simulink model "V2x_TC_IntelligentTrafficSigns_cs"  (Simulink 7.6)
settings: Solver=FixedStepDiscrete  SolverMode=SingleTasking  StartTime=0.0  StopTime=66  FixedStep=1/100  RelTol=1e-3
PreLoadFcn:
  | check_NUTS_defined
  | 
  | assignin('base',['model_closed_' bdroot],false);addpath('../includes_V2x');
InitFcn:
  | PreScanExperimentInitFcn(bdroot)
StopFcn:
  | PreScanExperimentStopFcn(bdroot);
  | 
undefined symbols (not defined in the file or in its folder): acceleration, actorID, actorType, DiscIntNeverNeededParam, GPS_alt, GPS_lat, GPS_long, lightBarInUse, messageID, Newton, publicActorType, Raphson, rotZ, sigbuilder_block, sireneInUse, trafficLightPriority, turnAdvice, velocity, x_ini_VRML, y_ini_VRML, yawRate, z_ini_VRML
libraries: simulink x111, PreScanSimulinkLibrary x7, simulink_extras x4, PreScanUsefulBlocks x3
(part 14 of 16)

== system V2x_TC_IntelligentTrafficSigns_cs/TS_v50_3/Infrastructure CAM generator/set CAM signals ==
  CAM generation signal : Inport
  warning : Inport  Port=2
  messageID : Inport  Port=3
  actorID : Inport  Port=4
  actorType : Inport  Port=5
  trafficLightPriority : Inport  Port=6
  GPS_lat : Inport  Port=7
  GPS_long : Inport  Port=8
  GPS_alt : Inport  Port=9
  rotZ : Inport  Port=10
  Mux : Mux  Inputs=9
  Switch : Switch  Criteria=u2 > Threshold  Threshold=0.5
  Unit Delay : UnitDelay  SampleTime=-1
  CAM_signals : Outport
  wires:
  rotZ/1 -> Mux/9
  GPS_alt/1 -> Mux/8
  GPS_long/1 -> Mux/7
  GPS_lat/1 -> Mux/6
  trafficLightPriority/1 -> Mux/5
  actorType/1 -> Mux/4
  actorID/1 -> Mux/3
  messageID/1 -> Mux/2
  warning/1 -> Mux/1
  Unit Delay/1 -> Switch/3
  Switch/1 -> CAM_signals/1, Unit Delay/1
  Mux/1 -> Switch/1
  CAM generation signal/1 -> Switch/2

== system V2x_TC_IntelligentTrafficSigns_cs/TS_v80_1/Infrastructure CAM generator/CAM generation ==
  Enable : EnablePort  StatesWhenEnabling=reset  PropagateVarSize=During execution
  Add : Sum  Inputs=+-
  Data Store Memory : DataStoreMemory  DataStoreName=CAM_time
  Data Store Read : DataStoreRead  DataStoreName=CAM_time  SampleTime=0.01
  Digital Clock : DigitalClock  SampleTime=0.01
  Merge : Merge
  if : If  IfExpression=u1 >= 1
  no : Subsystem  TreatAsAtomicUnit=on
  yes : Subsystem  TreatAsAtomicUnit=on
  CAN generation signal : Outport  OutputWhenDisabled=reset  InitialOutput=0
  wires:
  if/2 -> no/Action
  Merge/1 -> CAN generation signal/1
  no/1(Out1) -> Merge/2
  yes/1(Out1) -> Merge/1
  if/1 -> yes/Action
  Add/1 -> if/1
  Data Store Read/1 -> Add/2
  Digital Clock/1 -> Add/1

== system V2x_TC_IntelligentTrafficSigns_cs/TS_v80_1/Infrastructure CAM generator/Enabled Subsystem ==
  CAM_sig : Inport
  Enable : EnablePort  PropagateVarSize=During execution
  CAM_signals : Outport  OutputWhenDisabled=reset  InitialOutput=0
  wires:
  CAM_sig/1 -> CAM_signals/1

== system V2x_TC_IntelligentTrafficSigns_cs/TS_v80_1/Infrastructure CAM generator/coder ==
  R : Inport
  O : Inport  Port=2
  G : Inport  Port=3
  Add : Sum  Inputs=+++
  Gain : Gain  Gain=2
  Gain1 : Gain  Gain=3
  trafficLightPriority : Outport
  wires:
  Gain/1 -> Add/2
  Gain1/1 -> Add/3
  R/1 -> Add/1
  O/1 -> Gain/1
  Add/1 -> trafficLightPriority/1
  G/1 -> Gain1/1

== system V2x_TC_IntelligentTrafficSigns_cs/TS_v80_1/Infrastructure CAM generator/impulse generator ==
  CAM_time_step : Inport
  Add : Sum
  Compare To Zero : simulink/Logic and Bit Operations/Compare To Zero  SystemSampleTime=-1  relop=>  LogicOutDataTypeMode=boolean  ZeroCross=off
  Data Type Conversion : DataTypeConversion  OutDataTypeStr=boolean
  Detect Change : simulink/Logic and Bit Operations/Detect Change  SystemSampleTime=-1  vinit=0
  Digital Clock1 : DigitalClock  SampleTime=0.01
  Divide : Product  Inputs=*/
  Gain : Gain  Gain=pi
  Product : Product  RndMeth=Zero
  Trigonometric Function : Trigonometry
  constant : Constant
  experiment time step : Constant  Value=0.01  SampleTime=0
  enable : Outport
  wires:
  CAM_time_step/1 -> Divide/2
  Data Type Conversion/1 -> enable/1
  experiment time step/1 -> Add/2
  Add/1 -> Trigonometric Function/1
  Divide/1 -> Gain/1
  constant/1 -> Divide/1
  Product/1 -> Add/1
  Detect Change/1 -> Data Type Conversion/1
  Compare To Zero/1 -> Detect Change/1
  Trigonometric Function/1 -> Compare To Zero/1
  Gain/1 -> Product/2
  Digital Clock1/1 -> Product/1

== system V2x_TC_IntelligentTrafficSigns_cs/TS_v80_1/Infrastructure CAM generator/impulse generator1 ==
  CAM_time_step : Inport
  Add : Sum
  Compare To Zero : simulink/Logic and Bit Operations/Compare To Zero  SystemSampleTime=-1  relop=>  LogicOutDataTypeMode=boolean  ZeroCross=off
  Data Type Conversion : DataTypeConversion  OutDataTypeStr=boolean
  Detect Change : simulink/Logic and Bit Operations/Detect Change  SystemSampleTime=-1  vinit=0
  Digital Clock1 : DigitalClock  SampleTime=0.01
  Divide : Product  Inputs=*/
  Gain : Gain  Gain=pi
  Product : Product  RndMeth=Zero
  Trigonometric Function : Trigonometry
  constant : Constant
  experiment time step : Constant  Value=0.01  SampleTime=0
  enable : Outport
  wires:
  Digital Clock1/1 -> Product/1
  Gain/1 -> Product/2
  Trigonometric Function/1 -> Compare To Zero/1
  Compare To Zero/1 -> Detect Change/1
  Detect Change/1 -> Data Type Conversion/1
  Product/1 -> Add/1
  constant/1 -> Divide/1
  Divide/1 -> Gain/1
  Add/1 -> Trigonometric Function/1
  experiment time step/1 -> Add/2
  Data Type Conversion/1 -> enable/1
  CAM_time_step/1 -> Divide/2

== system V2x_TC_IntelligentTrafficSigns_cs/TS_v80_1/Infrastructure CAM generator/set CAM signals ==
  CAM generation signal : Inport
  warning : Inport  Port=2
  messageID : Inport  Port=3
  actorID : Inport  Port=4
  actorType : Inport  Port=5
  trafficLightPriority : Inport  Port=6
  GPS_lat : Inport  Port=7
  GPS_long : Inport  Port=8
  GPS_alt : Inport  Port=9
  rotZ : Inport  Port=10
  Mux : Mux  Inputs=9
  Switch : Switch  Criteria=u2 > Threshold  Threshold=0.5
  Unit Delay : UnitDelay  SampleTime=-1
  CAM_signals : Outport
  wires:
  rotZ/1 -> Mux/9
  GPS_alt/1 -> Mux/8
  GPS_long/1 -> Mux/7
  GPS_lat/1 -> Mux/6
  trafficLightPriority/1 -> Mux/5
  actorType/1 -> Mux/4
  actorID/1 -> Mux/3
  messageID/1 -> Mux/2
  warning/1 -> Mux/1
  Unit Delay/1 -> Switch/3
  Switch/1 -> CAM_signals/1, Unit Delay/1
  Mux/1 -> Switch/1
  CAM generation signal/1 -> Switch/2

== system V2x_TC_IntelligentTrafficSigns_cs/TS_v80_3/Infrastructure CAM generator/CAM generation ==
  Enable : EnablePort  StatesWhenEnabling=reset  PropagateVarSize=During execution
  Add : Sum  Inputs=+-
  Data Store Memory : DataStoreMemory  DataStoreName=CAM_time
  Data Store Read : DataStoreRead  DataStoreName=CAM_time  SampleTime=0.01
  Digital Clock : DigitalClock  SampleTime=0.01
  Merge : Merge
  if : If  IfExpression=u1 >= 1
  no : Subsystem  TreatAsAtomicUnit=on
  yes : Subsystem  TreatAsAtomicUnit=on
  CAN generation signal : Outport  OutputWhenDisabled=reset  InitialOutput=0
  wires:
  if/2 -> no/Action
  Merge/1 -> CAN generation signal/1
  no/1(Out1) -> Merge/2
  yes/1(Out1) -> Merge/1
  if/1 -> yes/Action
  Add/1 -> if/1
  Data Store Read/1 -> Add/2
  Digital Clock/1 -> Add/1

== system V2x_TC_IntelligentTrafficSigns_cs/TS_v80_3/Infrastructure CAM generator/Enabled Subsystem ==
  CAM_sig : Inport
  Enable : EnablePort  PropagateVarSize=During execution
  CAM_signals : Outport  OutputWhenDisabled=reset  InitialOutput=0
  wires:
  CAM_sig/1 -> CAM_signals/1

== system V2x_TC_IntelligentTrafficSigns_cs/TS_v80_3/Infrastructure CAM generator/coder ==
  R : Inport
  O : Inport  Port=2
  G : Inport  Port=3
  Add : Sum  Inputs=+++
  Gain : Gain  Gain=2
  Gain1 : Gain  Gain=3
  trafficLightPriority : Outport
  wires:
  Gain/1 -> Add/2
  Gain1/1 -> Add/3
  R/1 -> Add/1
  O/1 -> Gain/1
  Add/1 -> trafficLightPriority/1
  G/1 -> Gain1/1

== system V2x_TC_IntelligentTrafficSigns_cs/TS_v80_3/Infrastructure CAM generator/impulse generator ==
  CAM_time_step : Inport
  Add : Sum
  Compare To Zero : simulink/Logic and Bit Operations/Compare To Zero  SystemSampleTime=-1  relop=>  LogicOutDataTypeMode=boolean  ZeroCross=off
  Data Type Conversion : DataTypeConversion  OutDataTypeStr=boolean
  Detect Change : simulink/Logic and Bit Operations/Detect Change  SystemSampleTime=-1  vinit=0
  Digital Clock1 : DigitalClock  SampleTime=0.01
  Divide : Product  Inputs=*/
  Gain : Gain  Gain=pi
  Product : Product  RndMeth=Zero
  Trigonometric Function : Trigonometry
  constant : Constant
  experiment time step : Constant  Value=0.01  SampleTime=0
  enable : Outport
  wires:
  CAM_time_step/1 -> Divide/2
  Data Type Conversion/1 -> enable/1
  experiment time step/1 -> Add/2
  Add/1 -> Trigonometric Function/1
  Divide/1 -> Gain/1
  constant/1 -> Divide/1
  Product/1 -> Add/1
  Detect Change/1 -> Data Type Conversion/1
  Compare To Zero/1 -> Detect Change/1
  Trigonometric Function/1 -> Compare To Zero/1
  Gain/1 -> Product/2
  Digital Clock1/1 -> Product/1

== system V2x_TC_IntelligentTrafficSigns_cs/TS_v80_3/Infrastructure CAM generator/impulse generator1 ==
  CAM_time_step : Inport
  Add : Sum
  Compare To Zero : simulink/Logic and Bit Operations/Compare To Zero  SystemSampleTime=-1  relop=>  LogicOutDataTypeMode=boolean  ZeroCross=off
  Data Type Conversion : DataTypeConversion  OutDataTypeStr=boolean
  Detect Change : simulink/Logic and Bit Operations/Detect Change  SystemSampleTime=-1  vinit=0
  Digital Clock1 : DigitalClock  SampleTime=0.01
  Divide : Product  Inputs=*/
  Gain : Gain  Gain=pi
  Product : Product  RndMeth=Zero
  Trigonometric Function : Trigonometry
  constant : Constant
  experiment time step : Constant  Value=0.01  SampleTime=0
  enable : Outport
  wires:
  Digital Clock1/1 -> Product/1
  Gain/1 -> Product/2
  Trigonometric Function/1 -> Compare To Zero/1
  Compare To Zero/1 -> Detect Change/1
  Detect Change/1 -> Data Type Conversion/1
  Product/1 -> Add/1
  constant/1 -> Divide/1
  Divide/1 -> Gain/1
  Add/1 -> Trigonometric Function/1
  experiment time step/1 -> Add/2
  Data Type Conversion/1 -> enable/1
  CAM_time_step/1 -> Divide/2

== system V2x_TC_IntelligentTrafficSigns_cs/TS_v80_3/Infrastructure CAM generator/set CAM signals ==
  CAM generation signal : Inport
  warning : Inport  Port=2
  messageID : Inport  Port=3
  actorID : Inport  Port=4
  actorType : Inport  Port=5
  trafficLightPriority : Inport  Port=6
  GPS_lat : Inport  Port=7
  GPS_long : Inport  Port=8
  GPS_alt : Inport  Port=9
  rotZ : Inport  Port=10
  Mux : Mux  Inputs=9
  Switch : Switch  Criteria=u2 > Threshold  Threshold=0.5
  Unit Delay : UnitDelay  SampleTime=-1
  CAM_signals : Outport
  wires:
  rotZ/1 -> Mux/9
  GPS_alt/1 -> Mux/8
  GPS_long/1 -> Mux/7
  GPS_lat/1 -> Mux/6
  trafficLightPriority/1 -> Mux/5
  actorType/1 -> Mux/4
  actorID/1 -> Mux/3
  messageID/1 -> Mux/2
  warning/1 -> Mux/1
  Unit Delay/1 -> Switch/3
  Switch/1 -> CAM_signals/1, Unit Delay/1
  Mux/1 -> Switch/1
  CAM generation signal/1 -> Switch/2

== system V2x_TC_IntelligentTrafficSigns_cs/TS_v80_4/Infrastructure CAM generator/CAM generation ==
  Enable : EnablePort  StatesWhenEnabling=reset  PropagateVarSize=During execution
  Add : Sum  Inputs=+-
  Data Store Memory : DataStoreMemory  DataStoreName=CAM_time
  Data Store Read : DataStoreRead  DataStoreName=CAM_time  SampleTime=0.01
  Digital Clock : DigitalClock  SampleTime=0.01
  Merge : Merge
  if : If  IfExpression=u1 >= 1
  no : Subsystem  TreatAsAtomicUnit=on
  yes : Subsystem  TreatAsAtomicUnit=on
  CAN generation signal : Outport  OutputWhenDisabled=reset  InitialOutput=0
  wires:
  if/2 -> no/Action
  Merge/1 -> CAN generation signal/1
  no/1(Out1) -> Merge/2
  yes/1(Out1) -> Merge/1
  if/1 -> yes/Action
  Add/1 -> if/1
  Data Store Read/1 -> Add/2
  Digital Clock/1 -> Add/1

== system V2x_TC_IntelligentTrafficSigns_cs/TS_v80_4/Infrastructure CAM generator/Enabled Subsystem ==
  CAM_sig : Inport
  Enable : EnablePort  PropagateVarSize=During execution
  CAM_signals : Outport  OutputWhenDisabled=reset  InitialOutput=0
  wires:
  CAM_sig/1 -> CAM_signals/1

== system V2x_TC_IntelligentTrafficSigns_cs/TS_v80_4/Infrastructure CAM generator/coder ==
  R : Inport
  O : Inport  Port=2
  G : Inport  Port=3
  Add : Sum  Inputs=+++
  Gain : Gain  Gain=2
  Gain1 : Gain  Gain=3
  trafficLightPriority : Outport
  wires:
  Gain/1 -> Add/2
  Gain1/1 -> Add/3
  R/1 -> Add/1
  O/1 -> Gain/1
  Add/1 -> trafficLightPriority/1
  G/1 -> Gain1/1

== system V2x_TC_IntelligentTrafficSigns_cs/TS_v80_4/Infrastructure CAM generator/impulse generator ==
  CAM_time_step : Inport
  Add : Sum
  Compare To Zero : simulink/Logic and Bit Operations/Compare To Zero  SystemSampleTime=-1  relop=>  LogicOutDataTypeMode=boolean  ZeroCross=off
  Data Type Conversion : DataTypeConversion  OutDataTypeStr=boolean
  Detect Change : simulink/Logic and Bit Operations/Detect Change  SystemSampleTime=-1  vinit=0
  Digital Clock1 : DigitalClock  SampleTime=0.01
  Divide : Product  Inputs=*/
  Gain : Gain  Gain=pi
  Product : Product  RndMeth=Zero
  Trigonometric Function : Trigonometry
  constant : Constant
  experiment time step : Constant  Value=0.01  SampleTime=0
  enable : Outport
  wires:
  CAM_time_step/1 -> Divide/2
  Data Type Conversion/1 -> enable/1
  experiment time step/1 -> Add/2
  Add/1 -> Trigonometric Function/1
  Divide/1 -> Gain/1
  constant/1 -> Divide/1
  Product/1 -> Add/1
  Detect Change/1 -> Data Type Conversion/1
  Compare To Zero/1 -> Detect Change/1
  Trigonometric Function/1 -> Compare To Zero/1
  Gain/1 -> Product/2
  Digital Clock1/1 -> Product/1

== system V2x_TC_IntelligentTrafficSigns_cs/TS_v80_4/Infrastructure CAM generator/impulse generator1 ==
  CAM_time_step : Inport
  Add : Sum
  Compare To Zero : simulink/Logic and Bit Operations/Compare To Zero  SystemSampleTime=-1  relop=>  LogicOutDataTypeMode=boolean  ZeroCross=off
  Data Type Conversion : DataTypeConversion  OutDataTypeStr=boolean
  Detect Change : simulink/Logic and Bit Operations/Detect Change  SystemSampleTime=-1  vinit=0
  Digital Clock1 : DigitalClock  SampleTime=0.01
  Divide : Product  Inputs=*/
  Gain : Gain  Gain=pi
  Product : Product  RndMeth=Zero
  Trigonometric Function : Trigonometry
  constant : Constant
  experiment time step : Constant  Value=0.01  SampleTime=0
  enable : Outport
  wires:
  Digital Clock1/1 -> Product/1
  Gain/1 -> Product/2
  Trigonometric Function/1 -> Compare To Zero/1
  Compare To Zero/1 -> Detect Change/1
  Detect Change/1 -> Data Type Conversion/1
  Product/1 -> Add/1
  constant/1 -> Divide/1
  Divide/1 -> Gain/1
  Add/1 -> Trigonometric Function/1
  experiment time step/1 -> Add/2
  Data Type Conversion/1 -> enable/1
  CAM_time_step/1 -> Divide/2

== system V2x_TC_IntelligentTrafficSigns_cs/TS_v80_4/Infrastructure CAM generator/set CAM signals ==
  CAM generation signal : Inport
  warning : Inport  Port=2
  messageID : Inport  Port=3
  actorID : Inport  Port=4
  actorType : Inport  Port=5
  trafficLightPriority : Inport  Port=6
  GPS_lat : Inport  Port=7
  GPS_long : Inport  Port=8
  GPS_alt : Inport  Port=9
  rotZ : Inport  Port=10
  Mux : Mux  Inputs=9
  Switch : Switch  Criteria=u2 > Threshold  Threshold=0.5
  Unit Delay : UnitDelay  SampleTime=-1
  CAM_signals : Outport
  wires:
  rotZ/1 -> Mux/9
  GPS_alt/1 -> Mux/8
  GPS_long/1 -> Mux/7
  GPS_lat/1 -> Mux/6
  trafficLightPriority/1 -> Mux/5
  actorType/1 -> Mux/4
  actorID/1 -> Mux/3
  messageID/1 -> Mux/2
  warning/1 -> Mux/1
  Unit Delay/1 -> Switch/3
  Switch/1 -> CAM_signals/1, Unit Delay/1
  Mux/1 -> Switch/1
  CAM generation signal/1 -> Switch/2

== system V2x_TC_IntelligentTrafficSigns_cs/VISUWheels_BMW_X5_1/conv_RotWheelFL/RotMat2AxisAngle ==
  RotMat_Visu : Inport
  Data Type Conversion : DataTypeConversion
  Divide : Product  Inputs=*/
  Gain : Gain  Gain=2
  Mux : Mux  Inputs=3
  Mux1 : Mux  Inputs=2
  Saturation1 : Saturate  UpperLimit=3-0.001  LowerLimit=-1+0.001
  Trigonometric Function : Trigonometry
  U1 : Fcn  Expr=u[6]-u[8]
  U2 : Fcn  Expr=u[7]-u[3]
  U3 : Fcn  Expr=u[2]-u[4]
  U_Phi : Fcn  Expr=u[1]+u[5]+u[9]
  U_Phi1 : Fcn  Expr=acos((u(1)-1)/2)
  U_Phi2 : Fcn  Expr=acos((u(1)-1)/2)
  Angles_Visu : Outport
  wires:
  U_Phi2/1 -> Trigonometric Function/1
  Saturation1/1 -> U_Phi2/1
  U_Phi1/1 -> Data Type Conversion/1
  U_Phi/1 -> Saturation1/1, U_Phi1/1
  Divide/1 -> Mux1/1
  Mux1/1 -> Angles_Visu/1
  Gain/1 -> Divide/2
  Mux/1 -> Divide/1
  U3/1 -> Mux/3
  U2/1 -> Mux/2
  U1/1 -> Mux/1
  Trigonometric Function/1 -> Gain/1
  RotMat_Visu/1 -> U_Phi/1, U3/1, U2/1, U1/1
  Data Type Conversion/1 -> Mux1/2

== system V2x_TC_IntelligentTrafficSigns_cs/VISUWheels_BMW_X5_1/conv_RotWheelFR/RotMat2AxisAngle ==
  RotMat_Visu : Inport
  Data Type Conversion : DataTypeConversion
  Divide : Product  Inputs=*/
  Gain : Gain  Gain=2
  Mux : Mux  Inputs=3
  Mux1 : Mux  Inputs=2
  Saturation1 : Saturate  UpperLimit=3-0.001  LowerLimit=-1+0.001
  Trigonometric Function : Trigonometry
  U1 : Fcn  Expr=u[6]-u[8]
  U2 : Fcn  Expr=u[7]-u[3]
  U3 : Fcn  Expr=u[2]-u[4]
  U_Phi : Fcn  Expr=u[1]+u[5]+u[9]
  U_Phi1 : Fcn  Expr=acos((u(1)-1)/2)
  U_Phi2 : Fcn  Expr=acos((u(1)-1)/2)
  Angles_Visu : Outport
  wires:
  Data Type Conversion/1 -> Mux1/2
  RotMat_Visu/1 -> U1/1, U2/1, U3/1, U_Phi/1
  Trigonometric Function/1 -> Gain/1
  U1/1 -> Mux/1
  U2/1 -> Mux/2
  U3/1 -> Mux/3
  Mux/1 -> Divide/1
  Gain/1 -> Divide/2
  Mux1/1 -> Angles_Visu/1
  Divide/1 -> Mux1/1
  U_Phi/1 -> U_Phi1/1, Saturation1/1
  U_Phi1/1 -> Data Type Conversion/1
  Saturation1/1 -> U_Phi2/1
  U_Phi2/1 -> Trigonometric Function/1

== system V2x_TC_IntelligentTrafficSigns_cs/VISUWheels_BMW_X5_1/conv_RotWheelRL/RotMat2AxisAngle ==
  RotMat_Visu : Inport
  Data Type Conversion : DataTypeConversion
  Divide : Product  Inputs=*/
  Gain : Gain  Gain=2
  Mux : Mux  Inputs=3
  Mux1 : Mux  Inputs=2
  Saturation1 : Saturate  UpperLimit=3-0.001  LowerLimit=-1+0.001
  Trigonometric Function : Trigonometry
  U1 : Fcn  Expr=u[6]-u[8]
  U2 : Fcn  Expr=u[7]-u[3]
  U3 : Fcn  Expr=u[2]-u[4]
  U_Phi : Fcn  Expr=u[1]+u[5]+u[9]
  U_Phi1 : Fcn  Expr=acos((u(1)-1)/2)
  U_Phi2 : Fcn  Expr=acos((u(1)-1)/2)
  Angles_Visu : Outport
  wires:
  Data Type Conversion/1 -> Mux1/2
  RotMat_Visu/1 -> U1/1, U2/1, U3/1, U_Phi/1
  Trigonometric Function/1 -> Gain/1
  U1/1 -> Mux/1
  U2/1 -> Mux/2
  U3/1 -> Mux/3
  Mux/1 -> Divide/1
  Gain/1 -> Divide/2
  Mux1/1 -> Angles_Visu/1
  Divide/1 -> Mux1/1
  U_Phi/1 -> U_Phi1/1, Saturation1/1
  U_Phi1/1 -> Data Type Conversion/1
  Saturation1/1 -> U_Phi2/1
  U_Phi2/1 -> Trigonometric Function/1

== system V2x_TC_IntelligentTrafficSigns_cs/VISUWheels_BMW_X5_1/conv_RotWheelRR/RotMat2AxisAngle ==
  RotMat_Visu : Inport
  Data Type Conversion : DataTypeConversion
  Divide : Product  Inputs=*/
  Gain : Gain  Gain=2
  Mux : Mux  Inputs=3
  Mux1 : Mux  Inputs=2
  Saturation1 : Saturate  UpperLimit=3-0.001  LowerLimit=-1+0.001
  Trigonometric Function : Trigonometry
  U1 : Fcn  Expr=u[6]-u[8]
  U2 : Fcn  Expr=u[7]-u[3]
  U3 : Fcn  Expr=u[2]-u[4]
  U_Phi : Fcn  Expr=u[1]+u[5]+u[9]
  U_Phi1 : Fcn  Expr=acos((u(1)-1)/2)
  U_Phi2 : Fcn  Expr=acos((u(1)-1)/2)
  Angles_Visu : Outport
  wires:
  Data Type Conversion/1 -> Mux1/2
  RotMat_Visu/1 -> U1/1, U2/1, U3/1, U_Phi/1
  Trigonometric Function/1 -> Gain/1
  U1/1 -> Mux/1
  U2/1 -> Mux/2
  U3/1 -> Mux/3
  Mux/1 -> Divide/1
  Gain/1 -> Divide/2
  Mux1/1 -> Angles_Visu/1
  Divide/1 -> Mux1/1
  U_Phi/1 -> U_Phi1/1, Saturation1/1
  U_Phi1/1 -> Data Type Conversion/1
  Saturation1/1 -> U_Phi2/1
  U_Phi2/1 -> Trigonometric Function/1

== system V2x_TC_IntelligentTrafficSigns_cs/VISUWheels_BMW_X5_1/conv_TransWheelFL/pos_ini_Visu ==
  Constant : Constant  Value=x_ini_VRML
  Constant1 : Constant  Value=y_ini_VRML
  Constant2 : Constant  Value=z_ini_VRML
  Mux1 : Mux  Inputs=3
  pos_ini_Visu : Outport
  wires:
  Constant2/1 -> Mux1/3
  Constant1/1 -> Mux1/2
  Mux1/1 -> pos_ini_Visu/1
  Constant/1 -> Mux1/1

== system V2x_TC_IntelligentTrafficSigns_cs/VISUWheels_BMW_X5_1/conv_TransWheelFL/transISO2Visu ==
  transISO : Inport
  Demux : Demux  Outputs=3
  trans_x_Visu : Outport
  trans_y_Visu : Outport  Port=2
  trans_z_Visu : Outport  Port=3
  wires:
  Demux/3 -> trans_z_Visu/1
  transISO/1 -> Demux/1
  Demux/1 -> trans_x_Visu/1
  Demux/2 -> trans_y_Visu/1  "rot_y"

== system V2x_TC_IntelligentTrafficSigns_cs/VISUWheels_BMW_X5_1/conv_TransWheelFR/pos_ini_Visu ==
  Constant : Constant  Value=x_ini_VRML
  Constant1 : Constant  Value=y_ini_VRML
  Constant2 : Constant  Value=z_ini_VRML
  Mux1 : Mux  Inputs=3
  pos_ini_Visu : Outport
  wires:
  Constant/1 -> Mux1/1
  Mux1/1 -> pos_ini_Visu/1
  Constant1/1 -> Mux1/2
  Constant2/1 -> Mux1/3

== system V2x_TC_IntelligentTrafficSigns_cs/VISUWheels_BMW_X5_1/conv_TransWheelFR/transISO2Visu ==
  transISO : Inport
  Demux : Demux  Outputs=3
  trans_x_Visu : Outport
  trans_y_Visu : Outport  Port=2
  trans_z_Visu : Outport  Port=3
  wires:
  Demux/3 -> trans_z_Visu/1
  transISO/1 -> Demux/1
  Demux/1 -> trans_x_Visu/1
  Demux/2 -> trans_y_Visu/1  "rot_y"

== system V2x_TC_IntelligentTrafficSigns_cs/VISUWheels_BMW_X5_1/conv_TransWheelRL/pos_ini_Visu ==
  Constant : Constant  Value=x_ini_VRML
  Constant1 : Constant  Value=y_ini_VRML
  Constant2 : Constant  Value=z_ini_VRML
  Mux1 : Mux  Inputs=3
  pos_ini_Visu : Outport
  wires:
  Constant/1 -> Mux1/1
  Mux1/1 -> pos_ini_Visu/1
  Constant1/1 -> Mux1/2
  Constant2/1 -> Mux1/3

== system V2x_TC_IntelligentTrafficSigns_cs/VISUWheels_BMW_X5_1/conv_TransWheelRL/transISO2Visu ==
  transISO : Inport
  Demux : Demux  Outputs=3
  trans_x_Visu : Outport
  trans_y_Visu : Outport  Port=2
  trans_z_Visu : Outport  Port=3
  wires:
  Demux/3 -> trans_z_Visu/1
  transISO/1 -> Demux/1
  Demux/1 -> trans_x_Visu/1
  Demux/2 -> trans_y_Visu/1  "rot_y"

== system V2x_TC_IntelligentTrafficSigns_cs/VISUWheels_BMW_X5_1/conv_TransWheelRR/pos_ini_Visu ==
  Constant : Constant  Value=x_ini_VRML
  Constant1 : Constant  Value=y_ini_VRML
  Constant2 : Constant  Value=z_ini_VRML
  Mux1 : Mux  Inputs=3
  pos_ini_Visu : Outport
  wires:
  Constant/1 -> Mux1/1
  Mux1/1 -> pos_ini_Visu/1
  Constant1/1 -> Mux1/2
  Constant2/1 -> Mux1/3

== system V2x_TC_IntelligentTrafficSigns_cs/VISUWheels_BMW_X5_1/conv_TransWheelRR/transISO2Visu ==
  transISO : Inport
  Demux : Demux  Outputs=3
  trans_x_Visu : Outport
  trans_y_Visu : Outport  Port=2
  trans_z_Visu : Outport  Port=3
  wires:
  Demux/3 -> trans_z_Visu/1
  transISO/1 -> Demux/1
  Demux/1 -> trans_x_Visu/1
  Demux/2 -> trans_y_Visu/1  "rot_y"

== system V2x_TC_IntelligentTrafficSigns_cs/BMW_X5_1/Car_Rx_1_Demux/compas converter/cart2compas ==
  Angle [deg] : Inport
  Constant : Constant  Value=90
  Constant1 : Constant  Value=360
  Gain : Gain  Gain=1/360
  Gain1 : Gain  Gain=360
  Gain2 : Gain  Gain=-1
  Rounding Function : Rounding
  Sum : Sum  Inputs=|-+
  Sum1 : Sum  Inputs=-+|
  Sum2 : Sum  Inputs=++|
  heading [deg] : Outport
  wires:
  Gain/1 -> Rounding Function/1
  Rounding Function/1 -> Gain1/1
  Gain1/1 -> Sum/1
  Angle [deg]/1 -> Sum1/2
  Sum/1 -> Gain2/1
  Sum1/1 -> Gain/1, Sum/2
  Constant/1 -> Sum1/1
  Gain2/1 -> Sum2/2
  Constant1/1 -> Sum2/1
  Sum2/1 -> heading [deg]/1

== system V2x_TC_IntelligentTrafficSigns_cs/BMW_X5_1/Rx_Noise/For Iterator Subsystem1/con1 ==
  tmp_in : Inport
  i : Inport  Port=2
  CAM_in : Inport  Port=3
  Assignment : Assignment  IndexOptions=Index vector (port)  Indices=1  OutputSizes=1
  Bias1 : Bias  Bias=1
  Selector4 : Selector  NumberOfDimensions=2  IndexOptions=Index vector (port),Index vector (dialog)  Indices=[1 3],3  OutputSizes=1,1
  Selector5 : Selector  IndexOptions=Index vector (port)  Indices=[1 3]  OutputSizes=1
  Switch : Switch  Criteria=u2 ~= 0
  tmp_out : Outport
  wires:
  Selector4/1 -> Switch/2
  Assignment/1 -> Switch/1
  Selector5/1 -> Bias1/1
  Bias1/1 -> Assignment/2
  CAM_in/1 -> Selector4/1
  Switch/1 -> tmp_out/1
  tmp_in/1 -> Assignment/1, Selector5/1, Switch/3
  i/1 -> Selector4/2, Assignment/3, Selector5/2

== system V2x_TC_IntelligentTrafficSigns_cs/BMW_X5_1/Rx_Noise/For Iterator Subsystem1/con2 ==
  CAM_in : Inport
  i : Inport  Port=2
  NoMM : Inport  Port=3
  tmp_in : Inport  Port=4
  Assignment : Assignment  NumberOfDimensions=2  IndexOptions=Index vector (port),Assign all  Indices=1,1  OutputSizes=1,1
  Bias : Bias  Bias=1
  Compare To Zero : simulink/Logic and Bit Operations/Compare To Zero  SystemSampleTime=-1  relop=~=  LogicOutDataTypeMode=boolean
  Compare To Zero1 : simulink/Logic and Bit Operations/Compare To Zero  SystemSampleTime=-1  relop=~=  LogicOutDataTypeMode=boolean
  Constant : Constant  Value=0
  Logical Operator : Logic  AllPortsSameDT=off  OutDataTypeStr=boolean
  Math Function : Math  Operator=mod  SaturateOnIntegerOverflow=on
  Selector4 : Selector  NumberOfDimensions=2  IndexOptions=Index vector (port),Index vector (dialog)  Indices=[1 3],3  OutputSizes=1,1
  Selector5 : Selector  IndexOptions=Index vector (port)  Indices=[1 3]  OutputSizes=1
  Switch : Switch  Criteria=u2 ~= 0
  CAM_o : Outport
  wires:
  Switch/1 -> CAM_o/1
  CAM_in/1 -> Selector4/1, Switch/3, Assignment/1
  Selector4/1 -> Compare To Zero/1
  i/1 -> Selector4/2, Selector5/2, Assignment/3
  Compare To Zero/1 -> Logical Operator/1
  Logical Operator/1 -> Switch/2
  Compare To Zero1/1 -> Logical Operator/2
  Math Function/1 -> Compare To Zero1/1
  tmp_in/1 -> Selector5/1
  Selector5/1 -> Math Function/1
  NoMM/1 -> Bias/1
  Bias/1 -> Math Function/2
  Assignment/1 -> Switch/1
  Constant/1 -> Assignment/2

== system V2x_TC_IntelligentTrafficSigns_cs/BMW_X5_1/SELF_Demux/compas converter/cart2compas ==
  Angle [deg] : Inport
  Constant : Constant  Value=90
  Constant1 : Constant  Value=360
  Gain : Gain  Gain=1/360
  Gain1 : Gain  Gain=360
  Gain2 : Gain  Gain=-1
  Rounding Function : Rounding
  Sum : Sum  Inputs=|-+
  Sum1 : Sum  Inputs=-+|
  Sum2 : Sum  Inputs=++|
  heading [deg] : Outport
  wires:
  Sum2/1 -> heading [deg]/1
  Constant1/1 -> Sum2/1
  Gain2/1 -> Sum2/2
  Constant/1 -> Sum1/1
  Sum1/1 -> Sum/2, Gain/1
  Sum/1 -> Gain2/1
  Angle [deg]/1 -> Sum1/2
  Gain1/1 -> Sum/1
  Rounding Function/1 -> Gain1/1
  Gain/1 -> Rounding Function/1

== system V2x_TC_IntelligentTrafficSigns_cs/BMW_X5_1/Traffic Controller/Intersection warning controller/Enabled Subsystem ==
  crossingType : Inport
  hostTurnAdvice : Inport  Port=2
  hostRotZ : Inport  Port=3
  host GPS lat [deg] : Inport  Port=4
  host GPS long [deg] : Inport  Port=5
  host GPS alt [m] : Inport  Port=6
  GPS_lat : Inport  Port=7
  GPS_long : Inport  Port=8
  GPS_alt : Inport  Port=9
  actorID : Inport  Port=10
  targetVelocity : Inport  Port=11
  turnAdvice : Inport  Port=12
  rotZ : Inport  Port=13
  actorType : Inport  Port=14
  crossing detection range : Inport  Port=15
  Enable : EnablePort  PropagateVarSize=During execution
  Intersection : Subsystem
  sort using ID : Subsystem
  warning left lane : Outport
  warning straight lane : Outport  Port=2
  warning right lane : Outport  Port=3
  wires:
  hostRotZ/1 -> Intersection/1(hostRotZ)
  hostTurnAdvice/1 -> Intersection/2(hostTurnAdvice)
  host GPS lat [deg]/1 -> Intersection/3(host GPS lat [deg])
  host GPS long [deg]/1 -> Intersection/4(host GPS long [deg])
  host GPS alt [m]/1 -> Intersection/5(host GPS alt [m])
  actorID/1 -> sort using ID/1(IDs)
  GPS_lat/1 -> sort using ID/2(in1)
  GPS_long/1 -> sort using ID/3(in2)
  GPS_alt/1 -> sort using ID/4(in3)
  targetVelocity/1 -> sort using ID/5(in4)
  turnAdvice/1 -> sort using ID/6(in5)
  rotZ/1 -> sort using ID/7(in6)
  actorType/1 -> sort using ID/8(in7)
  crossingType/1 -> sort using ID/9(in8)
  sort using ID/1(Out1) -> Intersection/6(GPS_lat)
  sort using ID/2(Out2) -> Intersection/7(GPS_long)
  sort using ID/3(Out3) -> Intersection/8(GPS_alt)
  sort using ID/4(Out4) -> Intersection/9(targetVelocity)
  sort using ID/5(Out5) -> Intersection/10(turnAdvice)
  sort using ID/6(Out6) -> Intersection/11(rotZ)
  sort using ID/7(Out7) -> Intersection/12(actorType)
  sort using ID/8(Out8) -> Intersection/13(crossingType)
  Intersection/1(warning left lane) -> warning left lane/1
  Intersection/2(warning straight lane) -> warning straight lane/1
  Intersection/3(warning right lane) -> warning right lane/1
  crossing detection range/1 -> Intersection/14(crossing detection range)

== system V2x_TC_IntelligentTrafficSigns_cs/BMW_X5_1/Traffic Controller/Road accident detection controller/Compute distance to road accident ==
  conditions : Inport
  host_GPS lat [deg] : Inport  Port=2
  host_GPS lat [deg]1 : Inport  Port=3
  host_GPS alt [m] : Inport  Port=4
  GPS lat [deg] : Inport  Port=5
  GPS long [deg] : Inport  Port=6
  GPS alt [deg] : Inport  Port=7
  Enable : EnablePort  ShowOutputPort=on
  If : If  IfExpression=u1
  If Action Subsystem : Subsystem  TreatAsAtomicUnit=on
  If Action Subsystem1 : Subsystem  TreatAsAtomicUnit=on
  Merge : Merge
  RA_warning : Outport  OutputWhenDisabled=reset  InitialOutput=0
  RA_distance : Outport  Port=2  OutputWhenDisabled=reset  InitialOutput=0
  wires:
  GPS alt [deg]/1 -> If Action Subsystem/4(GPS alt1)
  If/2 -> If Action Subsystem1/Action
  If Action Subsystem1/1(distance) -> Merge/2
  If Action Subsystem/1(distance) -> Merge/1
  GPS long [deg]/1 -> If Action Subsystem/3(GPS long1)
  GPS lat [deg]/1 -> If Action Subsystem/2(GPS lat1)
  If/1 -> If Action Subsystem/Action
  host_GPS lat [deg]1/1 -> If Action Subsystem/6(host_GPS_long)
  host_GPS lat [deg]/1 -> If Action Subsystem/5(host_GPS_lat)
  host_GPS alt [m]/1 -> If Action Subsystem/7(host_GPS_alt)
  Enable/1 -> If/1, RA_warning/1
  conditions/1 -> If Action Subsystem/1(conditions)
  Merge/1 -> RA_distance/1

== system V2x_TC_IntelligentTrafficSigns_cs/BMW_X5_1/Traffic Controller/Road accident detection controller/distance hold ==
  dist : Inport
  Constant : Constant  Value=0
  Discrete FIR Filter : DiscreteFir  NumCoeffs=0.1 * ones(1,10)
  Memory : Memory
  Switch : Switch  Criteria=u2 > Threshold
  Switch1 : Switch  Criteria=u2 ~= 0
  distance : Outport
  wires:
  dist/1 -> Switch/2, Switch/1, Discrete FIR Filter/1
  Switch/1 -> Memory/1, Switch1/1
  Memory/1 -> Switch/3
  Discrete FIR Filter/1 -> Switch1/2
  Constant/1 -> Switch1/3
  Switch1/1 -> distance/1

== system V2x_TC_IntelligentTrafficSigns_cs/BMW_X5_1/Traffic Controller/Road accident detection controller/warning hold ==
  warn : Inport
  Constant : Constant  Value=0
  Discrete FIR Filter : DiscreteFir  NumCoeffs=0.1 * ones(1,10)
  Memory : Memory
  Switch : Switch  Criteria=u2 > Threshold
  Switch1 : Switch  Criteria=u2 ~= 0
  warning : Outport
  wires:
  warn/1 -> Switch/2, Switch/1, Discrete FIR Filter/1
  Switch/1 -> Memory/1, Switch1/1
  Memory/1 -> Switch/3
  Discrete FIR Filter/1 -> Switch1/2
  Constant/1 -> Switch1/3
  Switch1/1 -> warning/1

== system V2x_TC_IntelligentTrafficSigns_cs/BMW_X5_1/Traffic Controller/Traffic signs and  traffic lights controller/Enabled Subsystem ==
  trafficSignType : Inport
  trafficLightsType : Inport  Port=2
  hostRotZ : Inport  Port=3
  hostVelocity : Inport  Port=4
  messageID : Inport  Port=5
  trafficLightPriority : Inport  Port=6
  rotZ : Inport  Port=7
  host GPS lat [deg] : Inport  Port=8
  host GPS long [deg] : Inport  Port=9
  host GPS alt [m] : Inport  Port=10
  GPS_lat : Inport  Port=11
  GPS_long : Inport  Port=12
  GPS_alt : Inport  Port=13
  Enable : EnablePort  StatesWhenEnabling=reset  PropagateVarSize=During execution
  GPS to XYZ difference (vectorized) : Subsystem
  Logical Operator : Logic  AllPortsSameDT=off  OutDataTypeStr=boolean
  Logical Operator1 : Logic  AllPortsSameDT=off  OutDataTypeStr=boolean
  Logical Operator2 : Logic  Inputs=4  AllPortsSameDT=off  OutDataTypeStr=boolean
  distance condition : Subsystem
  heading condition : Subsystem
  hostVelocity1 : InportShadow  Port=4
  major object selection lights : Subsystem
  major object selection signs : Subsystem
  orientation condition : Subsystem
  right road side condition : Subsystem
  signsWarning : Outport
  signsMessage : Outport  Port=2  InitialOutput=0
  lightsWarning : Outport  Port=3
  ligtsMessage : Outport  Port=4  InitialOutput=0
  ligtsPriority : Outport  Port=5  InitialOutput=0
  wires:
  GPS to XYZ difference (vectorized)/3(dY) -> right road side condition/3(dY), orientation condition/3(dY)
  right road side condition/1(orient_cond) -> Logical Operator2/4
  GPS to XYZ difference (vectorized)/1(dist_sq) -> distance condition/1(distance_squared)
  GPS to XYZ difference (vectorized)/2(dX) -> orientation condition/2(dX), right road side condition/2(dX)
  GPS to XYZ difference (vectorized)/4(dZ) -> orientation condition/4(dZ)
  GPS_alt/1 -> GPS to XYZ difference (vectorized)/6(alt)
  orientation condition/1(orient_cond) -> Logical Operator2/3
  GPS_long/1 -> GPS to XYZ difference (vectorized)/5(long)
  GPS_lat/1 -> GPS to XYZ difference (vectorized)/4(lat)
  host GPS alt [m]/1 -> GPS to XYZ difference (vectorized)/3(hostAlt)
  host GPS long [deg]/1 -> GPS to XYZ difference (vectorized)/2(hostLong)
  host GPS lat [deg]/1 -> GPS to XYZ difference (vectorized)/1(hostLat)
  hostVelocity1/1 -> major object selection signs/2(hostVelocity)
  major object selection lights/3(Priority) -> ligtsPriority/1
  major object selection lights/2(MessageID) -> ligtsMessage/1
  major object selection lights/1(Warning) -> lightsWarning/1
  trafficLightPriority/1 -> major object selection lights/3(traffic lights priorities)
  Logical Operator1/1 -> major object selection lights/1(conditions)
  major object selection signs/2(MessageID) -> signsMessage/1
  major object selection signs/1(Warning) -> signsWarning/1
  messageID/1 -> major object selection lights/2(messagesID), major object selection signs/3(messagesID)
  Logical Operator/1 -> major object selection signs/1(conditions)
  trafficLightsType/1 -> Logical Operator1/2
  trafficSignType/1 -> Logical Operator/2
  Logical Operator2/1 -> Logical Operator1/1, Logical Operator/1
  heading condition/1(head_cond) -> Logical Operator2/2
  distance condition/1(dist_cond) -> Logical Operator2/1
  hostRotZ/1 -> orientation condition/1(hostRotZ), right road side condition/1(hostRotZ), heading condition/1(hostRotZ)
  hostVelocity/1 -> distance condition/2(hostVelocity)
  rotZ/1 -> heading condition/2(rotZ)

== system V2x_TC_IntelligentTrafficSigns_cs/BMW_X5_1/Trajectories/ElapsedTrack1/MuxState ==
  xyzPos_[m] : Inport
  RPYAngle_[rad] : Inport  Port=2
  Demux : Demux  Outputs=3
  Discrete Derivative : simulink/Discrete/Discrete Derivative  SystemSampleTime=-1  gainval=1.0  ICPrevScaledInput=0.0  OutMin=[]  OutMax=[]  OutDataType=fixdt(1, 16)  OutScaling=2^-10  DoSatur=off
  Mux : Mux  Inputs=3
  Mux1 : Mux  Inputs=3
  Substitute 90 & 270 deg (and its plurals) : Subsystem
  State : Outport
  wires:
  Mux1/1 -> Mux/3
  Discrete Derivative/1 -> Mux/2
  RPYAngle_[rad]/1 -> Demux/1
  Demux/3 -> Mux1/3
  Demux/2 -> Substitute 90 & 270 deg (and its plurals)/1(Angle [rad])
  Demux/1 -> Mux1/1
  xyzPos_[m]/1 -> Discrete Derivative/1, Mux/1
  Mux/1 -> State/1
  Substitute 90 & 270 deg (and its plurals)/1(Subst Angle [rad]) -> Mux1/2

== system V2x_TC_IntelligentTrafficSigns_cs/BMW_X5_1/Trajectories/ElapsedTrack1/OffsetCor ==
  x pos : Inport
  y pos : Inport  Port=2
  z pos : Inport  Port=3
  R angle : Inport  Port=4
  P angle : Inport  Port=5
  Y angle : Inport  Port=6
  Offset : Inport  Port=7
  ApplyRotation : PreScanSimulinkLibrary/ApplyRotation  SystemSampleTime=-1
  Demux : Demux  Outputs=6
  Mux : Mux  Inputs=2
  Mux1 : Mux  Inputs=3
  Mux2 : Mux  Inputs=3
  Mux3 : Mux  Inputs=3
  Selector2 : Selector  InputPortWidth=6  IndexOptions=Index vector (dialog)  Indices=[4]  OutputSizes=1
  Selector3 : Selector  InputPortWidth=6  IndexOptions=Index vector (dialog)  Indices=[5]  OutputSizes=1
  Selector4 : Selector  InputPortWidth=6  IndexOptions=Index vector (dialog)  Indices=[6]  OutputSizes=1
  Sum : Sum  Inputs=|++
  x pos_cor : Outport
  y pos_cor : Outport  Port=2
  z pos_cor : Outport  Port=3
  R angle_cor : Outport  Port=4
  P angle_cor : Outport  Port=5
  Y angle_cor : Outport  Port=6
  wires:
  Mux3/1 -> ApplyRotation/1
  ApplyRotation/1 -> Mux/1
  Mux2/1 -> ApplyRotation/2
  Selector4/1 -> Mux2/3
  Selector3/1 -> Mux2/2
  Selector2/1 -> Mux2/1
  Mux1/1 -> Mux/2
  Y angle/1 -> Mux1/3
  P angle/1 -> Mux1/2
  R angle/1 -> Mux1/1
  Demux/1 -> x pos_cor/1
  Demux/2 -> y pos_cor/1
  Demux/3 -> z pos_cor/1
  Demux/4 -> R angle_cor/1
  Demux/5 -> P angle_cor/1
  Demux/6 -> Y angle_cor/1
  z pos/1 -> Mux3/3
  y pos/1 -> Mux3/2
  x pos/1 -> Mux3/1
  Offset/1 -> Selector4/1, Selector3/1, Selector2/1, Sum/2
  Mux/1 -> Sum/1
  Sum/1 -> Demux/1

== system V2x_TC_IntelligentTrafficSigns_cs/BMW_X5_1/Trajectories/Traj2State1/Traj2State2 ==
  traj : Inport
  MuxState : Subsystem
  Traj2MuxState_Ext : Subsystem
  state : Outport
  wires:
  Traj2MuxState_Ext/9(Y [rad]) -> MuxState/9(Y [rad])
  Traj2MuxState_Ext/8(P [rad]) -> MuxState/8(P [rad])
  Traj2MuxState_Ext/7(R [rad]) -> MuxState/7(R [rad])
  Traj2MuxState_Ext/6(dz [m/s]) -> MuxState/6(dz [m/s])
  Traj2MuxState_Ext/5(dy [m/s]) -> MuxState/5(dy [m/s])
  Traj2MuxState_Ext/4(dx [m/s]) -> MuxState/4(dx [m/s])
  Traj2MuxState_Ext/3(z [m]) -> MuxState/3(z [m])
  Traj2MuxState_Ext/2(y [m]) -> MuxState/2(y [m])
  Traj2MuxState_Ext/1(x [m]) -> MuxState/1(x [m])
  MuxState/1(state) -> state/1
  traj/1 -> Traj2MuxState_Ext/1(Traj)

== system V2x_TC_IntelligentTrafficSigns_cs/BMW_X5_1/vehicle CAM generator/CAM generation/maybe ==
  x [m] : Inport
  y [m] : Inport  Port=2
  z [m] : Inport  Port=3
  rotZ [deg] : Inport  Port=4
  velocity [m/s] : Inport  Port=5
  Action Port : ActionPort  PropagateVarSize=During execution  ActionType=else
  Add{,1..4} (5 alike) : Sum  Inputs=+-
  Add5 : Sum  Inputs=+++
  Compare To Constant : simulink/Logic and Bit Operations/Compare To Constant  SystemSampleTime=-1  relop=>  const=1  LogicOutDataTypeMode=uint8  ZeroCross=off
  Compare To Constant1 : simulink/Logic and Bit Operations/Compare To Constant  SystemSampleTime=-1  relop=>  const=4  LogicOutDataTypeMode=uint8  ZeroCross=off
  Compare To Constant2 : simulink/Logic and Bit Operations/Compare To Constant  SystemSampleTime=-1  relop=>  const=5^2  LogicOutDataTypeMode=uint8  ZeroCross=off
  Data Store Read : DataStoreRead  DataStoreName=CAM_velocity  SampleTime=0.01
  Data Store Read1 : DataStoreRead  DataStoreName=CAM_heading  SampleTime=0.01
  Data Store Read2 : DataStoreRead  DataStoreName=CAM_x  SampleTime=0.01
  Data Store Read3 : DataStoreRead  DataStoreName=CAM_y  SampleTime=0.01
  Data Store Read4 : DataStoreRead  DataStoreName=CAM_z  SampleTime=0.01
  If : If  IfExpression=u1
  If Action Subsystem : Subsystem  TreatAsAtomicUnit=on
  If Action Subsystem1 : Subsystem  TreatAsAtomicUnit=on
  Logical Operator : Logic  Operator=OR  Inputs=3  AllPortsSameDT=off  OutDataTypeStr=boolean
  Merge : Merge
  Product : Product  RndMeth=Zero
  Product1 : Product  RndMeth=Zero
  Product2 : Product  RndMeth=Zero
  rotZ [deg]1 : InportShadow  Port=4
  velocity [m/s]1 : InportShadow  Port=5
  x [m]1 : InportShadow  Port=1
  y [m]1 : InportShadow  Port=2
  z [m]1 : InportShadow  Port=3
  Out1 : Outport  OutputWhenDisabled=reset  InitialOutput=0
  wires:
  velocity [m/s]/1 -> Add/1
  Data Store Read/1 -> Add/2
  Add/1 -> Compare To Constant/1
  Add1/1 -> Compare To Constant1/1
  rotZ [deg]/1 -> Add1/1
  Data Store Read1/1 -> Add1/2
  x [m]/1 -> Add3/1
  Data Store Read2/1 -> Add3/2
  y [m]/1 -> Add2/1
  Data Store Read3/1 -> Add2/2
  z [m]/1 -> Add4/1
  Data Store Read4/1 -> Add4/2
  Add4/1 -> Product2/2, Product2/1
  Add2/1 -> Product1/2, Product1/1
  Add3/1 -> Product/2, Product/1
  Product/1 -> Add5/1
  Product1/1 -> Add5/2
  Product2/1 -> Add5/3
  Add5/1 -> Compare To Constant2/1
  Compare To Constant/1 -> Logical Operator/1
  Compare To Constant1/1 -> Logical Operator/2
  Compare To Constant2/1 -> Logical Operator/3
  Logical Operator/1 -> If/1
  If/1 -> If Action Subsystem/Action
  If/2 -> If Action Subsystem1/Action
  If Action Subsystem/1(Out1) -> Merge/1
  If Action Subsystem1/1(Out1) -> Merge/2
  Merge/1 -> Out1/1
  x [m]1/1 -> If Action Subsystem/1(x [m])
  y [m]1/1 -> If Action Subsystem/2(y [m])
  z [m]1/1 -> If Action Subsystem/3(z [m])
  rotZ [deg]1/1 -> If Action Subsystem/4(rotZ [deg])
  velocity [m/s]1/1 -> If Action Subsystem/5(velocity [m/s])

== system V2x_TC_IntelligentTrafficSigns_cs/BMW_X5_1/vehicle CAM generator/CAM generation/no ==
  Action Port : ActionPort  PropagateVarSize=During execution  ActionType=elseif
  Constant : Constant  Value=0
  Out1 : Outport  OutputWhenDisabled=reset  InitialOutput=0
  wires:
  Constant/1 -> Out1/1

== system V2x_TC_IntelligentTrafficSigns_cs/BMW_X5_1/vehicle CAM generator/CAM generation/yes ==
  x [m] : Inport
  y [m] : Inport  Port=2
  z [m] : Inport  Port=3
  rotZ [deg] : Inport  Port=4
  velocity [m/s] : Inport  Port=5
  Action Port : ActionPort  PropagateVarSize=During execution  ActionType=then
  Constant : Constant
  Data Store Write : DataStoreWrite  DataStoreName=CAM_velocity
  Data Store Write1 : DataStoreWrite  DataStoreName=CAM_heading
  Data Store Write2 : DataStoreWrite  DataStoreName=CAM_x
  Data Store Write3 : DataStoreWrite  DataStoreName=CAM_y
  Data Store Write4 : DataStoreWrite  DataStoreName=CAM_z
  Data Store Write5 : DataStoreWrite  DataStoreName=CAM_time
  Digital Clock1 : DigitalClock  SampleTime=0.01
  Out1 : Outport  OutputWhenDisabled=reset  InitialOutput=0
  wires:
  Constant/1 -> Out1/1
  velocity [m/s]/1 -> Data Store Write/1
  rotZ [deg]/1 -> Data Store Write1/1
  x [m]/1 -> Data Store Write2/1
  y [m]/1 -> Data Store Write3/1
  z [m]/1 -> Data Store Write4/1
  Digital Clock1/1 -> Data Store Write5/1

== system V2x_TC_IntelligentTrafficSigns_cs/CameraTrolley/SELF_Demux/compas converter/cart2compas ==
  Angle [deg] : Inport
  Constant : Constant  Value=90
  Constant1 : Constant  Value=360
  Gain : Gain  Gain=1/360
  Gain1 : Gain  Gain=360
  Gain2 : Gain  Gain=-1
  Rounding Function : Rounding
  Sum : Sum  Inputs=|-+
  Sum1 : Sum  Inputs=-+|
  Sum2 : Sum  Inputs=++|
  heading [deg] : Outport
  wires:
  Sum2/1 -> heading [deg]/1
  Constant1/1 -> Sum2/1
  Gain2/1 -> Sum2/2
  Constant/1 -> Sum1/1
  Sum1/1 -> Sum/2, Gain/1
  Sum/1 -> Gain2/1
  Angle [deg]/1 -> Sum1/2
  Gain1/1 -> Sum/1
  Rounding Function/1 -> Gain1/1
  Gain/1 -> Rounding Function/1

== system V2x_TC_IntelligentTrafficSigns_cs/TS_Lcurve_R14/Infrastructure CAM generator/CAM generation/no ==
  Action Port : ActionPort  PropagateVarSize=During execution  ActionType=else
  Constant : Constant  Value=0
  Out1 : Outport  OutputWhenDisabled=reset  InitialOutput=0
  wires:
  Constant/1 -> Out1/1

== system V2x_TC_IntelligentTrafficSigns_cs/TS_Lcurve_R14/Infrastructure CAM generator/CAM generation/yes ==
  Action Port : ActionPort  PropagateVarSize=During execution  ActionType=then
  Constant : Constant
  Data Store Write5 : DataStoreWrite  DataStoreName=CAM_time
  Digital Clock1 : DigitalClock  SampleTime=0.01
  Out1 : Outport  OutputWhenDisabled=reset  InitialOutput=0
  wires:
  Digital Clock1/1 -> Data Store Write5/1
  Constant/1 -> Out1/1

== system V2x_TC_IntelligentTrafficSigns_cs/TS_Lcurve_R25/Infrastructure CAM generator/CAM generation/no ==
  Action Port : ActionPort  PropagateVarSize=During execution  ActionType=else
  Constant : Constant  Value=0
  Out1 : Outport  OutputWhenDisabled=reset  InitialOutput=0
  wires:
  Constant/1 -> Out1/1

== system V2x_TC_IntelligentTrafficSigns_cs/TS_Lcurve_R25/Infrastructure CAM generator/CAM generation/yes ==
  Action Port : ActionPort  PropagateVarSize=During execution  ActionType=then
  Constant : Constant
  Data Store Write5 : DataStoreWrite  DataStoreName=CAM_time
  Digital Clock1 : DigitalClock  SampleTime=0.01
  Out1 : Outport  OutputWhenDisabled=reset  InitialOutput=0
  wires:
  Digital Clock1/1 -> Data Store Write5/1
  Constant/1 -> Out1/1

== system V2x_TC_IntelligentTrafficSigns_cs/TS_Lcurve_R39/Infrastructure CAM generator/CAM generation/no ==
  Action Port : ActionPort  PropagateVarSize=During execution  ActionType=else
  Constant : Constant  Value=0
  Out1 : Outport  OutputWhenDisabled=reset  InitialOutput=0
  wires:
  Constant/1 -> Out1/1

== system V2x_TC_IntelligentTrafficSigns_cs/TS_Lcurve_R39/Infrastructure CAM generator/CAM generation/yes ==
  Action Port : ActionPort  PropagateVarSize=During execution  ActionType=then
  Constant : Constant
  Data Store Write5 : DataStoreWrite  DataStoreName=CAM_time
  Digital Clock1 : DigitalClock  SampleTime=0.01
  Out1 : Outport  OutputWhenDisabled=reset  InitialOutput=0
  wires:
  Digital Clock1/1 -> Data Store Write5/1
  Constant/1 -> Out1/1

== system V2x_TC_IntelligentTrafficSigns_cs/TS_Lcurve_R55/Infrastructure CAM generator/CAM generation/no ==
  Action Port : ActionPort  PropagateVarSize=During execution  ActionType=else
  Constant : Constant  Value=0
  Out1 : Outport  OutputWhenDisabled=reset  InitialOutput=0
  wires:
  Constant/1 -> Out1/1

== system V2x_TC_IntelligentTrafficSigns_cs/TS_Lcurve_R55/Infrastructure CAM generator/CAM generation/yes ==
  Action Port : ActionPort  PropagateVarSize=During execution  ActionType=then
  Constant : Constant
  Data Store Write5 : DataStoreWrite  DataStoreName=CAM_time
  Digital Clock1 : DigitalClock  SampleTime=0.01
  Out1 : Outport  OutputWhenDisabled=reset  InitialOutput=0
  wires:
  Digital Clock1/1 -> Data Store Write5/1
  Constant/1 -> Out1/1

== system V2x_TC_IntelligentTrafficSigns_cs/TS_Rcurve_R14/Infrastructure CAM generator/CAM generation/no ==
  Action Port : ActionPort  PropagateVarSize=During execution  ActionType=else
  Constant : Constant  Value=0
  Out1 : Outport  OutputWhenDisabled=reset  InitialOutput=0
  wires:
  Constant/1 -> Out1/1

== system V2x_TC_IntelligentTrafficSigns_cs/TS_Rcurve_R14/Infrastructure CAM generator/CAM generation/yes ==
  Action Port : ActionPort  PropagateVarSize=During execution  ActionType=then
  Constant : Constant
  Data Store Write5 : DataStoreWrite  DataStoreName=CAM_time
  Digital Clock1 : DigitalClock  SampleTime=0.01
  Out1 : Outport  OutputWhenDisabled=reset  InitialOutput=0
  wires:
  Digital Clock1/1 -> Data Store Write5/1
  Constant/1 -> Out1/1

== system V2x_TC_IntelligentTrafficSigns_cs/TS_Rcurve_R25/Infrastructure CAM generator/CAM generation/no ==
  Action Port : ActionPort  PropagateVarSize=During execution  ActionType=else
  Constant : Constant  Value=0
  Out1 : Outport  OutputWhenDisabled=reset  InitialOutput=0
  wires:
  Constant/1 -> Out1/1

== system V2x_TC_IntelligentTrafficSigns_cs/TS_Rcurve_R25/Infrastructure CAM generator/CAM generation/yes ==
  Action Port : ActionPort  PropagateVarSize=During execution  ActionType=then
  Constant : Constant
  Data Store Write5 : DataStoreWrite  DataStoreName=CAM_time
  Digital Clock1 : DigitalClock  SampleTime=0.01
  Out1 : Outport  OutputWhenDisabled=reset  InitialOutput=0
  wires:
  Digital Clock1/1 -> Data Store Write5/1
  Constant/1 -> Out1/1

== system V2x_TC_IntelligentTrafficSigns_cs/TS_Rcurve_R39/Infrastructure CAM generator/CAM generation/no ==
  Action Port : ActionPort  PropagateVarSize=During execution  ActionType=else
  Constant : Constant  Value=0
  Out1 : Outport  OutputWhenDisabled=reset  InitialOutput=0
  wires:
  Constant/1 -> Out1/1

== system V2x_TC_IntelligentTrafficSigns_cs/TS_Rcurve_R39/Infrastructure CAM generator/CAM generation/yes ==
  Action Port : ActionPort  PropagateVarSize=During execution  ActionType=then
  Constant : Constant
  Data Store Write5 : DataStoreWrite  DataStoreName=CAM_time
  Digital Clock1 : DigitalClock  SampleTime=0.01
  Out1 : Outport  OutputWhenDisabled=reset  InitialOutput=0
  wires:
  Digital Clock1/1 -> Data Store Write5/1
  Constant/1 -> Out1/1

== system V2x_TC_IntelligentTrafficSigns_cs/TS_Rcurve_R55/Infrastructure CAM generator/CAM generation/no ==
  Action Port : ActionPort  PropagateVarSize=During execution  ActionType=else
  Constant : Constant  Value=0
  Out1 : Outport  OutputWhenDisabled=reset  InitialOutput=0
  wires:
  Constant/1 -> Out1/1

== system V2x_TC_IntelligentTrafficSigns_cs/TS_Rcurve_R55/Infrastructure CAM generator/CAM generation/yes ==
  Action Port : ActionPort  PropagateVarSize=During execution  ActionType=then
  Constant : Constant
  Data Store Write5 : DataStoreWrite  DataStoreName=CAM_time
  Digital Clock1 : DigitalClock  SampleTime=0.01
  Out1 : Outport  OutputWhenDisabled=reset  InitialOutput=0
  wires:
  Digital Clock1/1 -> Data Store Write5/1
  Constant/1 -> Out1/1

== system V2x_TC_IntelligentTrafficSigns_cs/TS_Tcross_1/Infrastructure CAM generator/CAM generation/no ==
  Action Port : ActionPort  PropagateVarSize=During execution  ActionType=else
  Constant : Constant  Value=0
  Out1 : Outport  OutputWhenDisabled=reset  InitialOutput=0
  wires:
  Constant/1 -> Out1/1

== system V2x_TC_IntelligentTrafficSigns_cs/TS_Tcross_1/Infrastructure CAM generator/CAM generation/yes ==
  Action Port : ActionPort  PropagateVarSize=During execution  ActionType=then
  Constant : Constant
  Data Store Write5 : DataStoreWrite  DataStoreName=CAM_time
  Digital Clock1 : DigitalClock  SampleTime=0.01
  Out1 : Outport  OutputWhenDisabled=reset  InitialOutput=0
  wires:
  Digital Clock1/1 -> Data Store Write5/1
  Constant/1 -> Out1/1
